simulink model "CCMVgaMotor"  (R2022b)
settings: Solver=FixedStepDiscrete  StartTime=0.0  StopTime=10.0  FixedStep=0.01  RelTol=1e-3
model workspace (MAT data in the model file):
  NvMBlockVgaMtrCalData : Simulink.Signal (value not decoded)
  NvMBlockVgaMtrCalDataInit : Simulink.Parameter (value not decoded)
  VgaWakeUpFlag : Simulink.Signal (value not decoded)
symbols that are objects in the model workspace (value not decoded): NvMBlockVgaMtrCalDataInit
undefined symbols (not defined in the file or in its folder): ComChlType, DioChannelType, LinFrameType, NetworkChlType, NvMBlockId, P_DioLevelType, P_LinIfSchHandleType, P_Std_ReturnType, PowerChlType, VGAChlType
(part 4 of 4)

== system CCMVgaMotor/VgaCaliServive ==
  VgaCaliServive_SetAllCalibrate_sys : Subsystem
  VgaCaliServive_SetAllParaInit_sys : Subsystem
  VgaCaliServive_SetMotorCalibrateNew_sys : Subsystem
  VgaCaliServive_SetMotorCalibrate_sys : Subsystem
  VgaCaliServive_SetMotorParaInit_sys : Subsystem

== system CCMVgaMotor/VgaDtcService ==
  VgaDtcService_GetElecErr_sys : Subsystem
  VgaDtcService_GetRecErr_sys : Subsystem
  VgaDtcService_GetStallErr_sys : Subsystem
  VgaDtcService_GetTempErr_sys : Subsystem
  VgaDtcService_GetVoltErr_sys : Subsystem

== system CCMVgaMotor/VgaIOCtrlService ==
  VgaIOCtrlService_FreezeMotorCurrentPosition_sys : Subsystem
  VgaIOCtrlService_MotorReturnToECU_sys : Subsystem
  VgaIOCtrlService_MotorShortTermAdj_sys : Subsystem

== system CCMVgaMotor/VgaNvmService ==
  VgaNvmService_ClerarNvmMotorData_sys : Subsystem

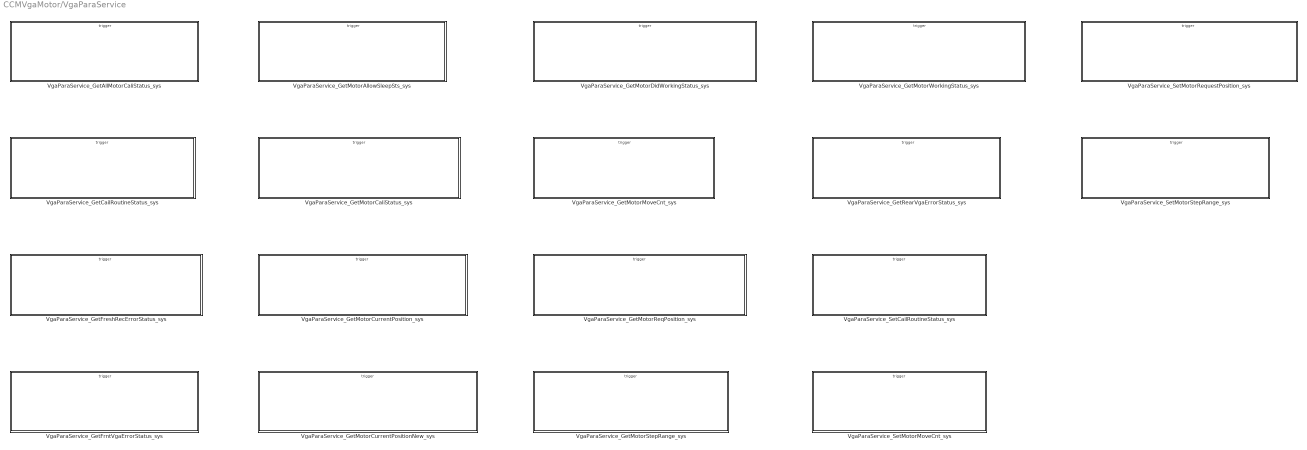
== system CCMVgaMotor/VgaParaService ==
  VgaParaService_GetAllMotorCaliStatus_sys : Subsystem
  VgaParaService_GetCailRoutineStatus_sys : Subsystem
  VgaParaService_GetFreshRecErrorStatus_sys : Subsystem
  VgaParaService_GetFrntVgaErrorStatus_sys : Subsystem
  VgaParaService_GetMotorAllowSleepSts_sys : Subsystem
  VgaParaService_GetMotorCaliStatus_sys : Subsystem
  VgaParaService_GetMotorCurrentPositionNew_sys : Subsystem
  VgaParaService_GetMotorCurrentPosition_sys : Subsystem
  VgaParaService_GetMotorDidWorkingStatus_sys : Subsystem
  VgaParaService_GetMotorMoveCnt_sys : Subsystem
  VgaParaService_GetMotorReqPosition_sys : Subsystem
  VgaParaService_GetMotorStepRange_sys : Subsystem
  VgaParaService_GetMotorWorkingStatus_sys : Subsystem
  VgaParaService_GetRearVgaErrorStatus_sys : Subsystem
  VgaParaService_SetCailRoutineStatus_sys : Subsystem
  VgaParaService_SetMotorMoveCnt_sys : Subsystem
  VgaParaService_SetMotorRequestPosition_sys : Subsystem
  VgaParaService_SetMotorStepRange_sys : Subsystem

== system CCMVgaMotor/VgaCaliServive/VgaCaliServive_SetAllCalibrate_sys ==
  function : TriggerPort  TriggerType=function-call  IsSimulinkFunction=on  FunctionName=VgaCaliServive_SetAllCalibrate  VariantControl=(inherit)
  Ground_1 : Ground
  PA_NvMBlockVgaMtrCalDataInit : Constant  Value=NvMBlockVgaMtrCalDataInit
  Terminator_1 : Terminator
  ERR : ArgOut  OutDataTypeStr=Std_ReturnType  ArgumentName=ERR
  wires:
  PA_NvMBlockVgaMtrCalDataInit/1 -> Terminator_1/1
  Ground_1/1 -> ERR/1

== system CCMVgaMotor/VgaCaliServive/VgaCaliServive_SetAllParaInit_sys ==
  function : TriggerPort  TriggerType=function-call  IsSimulinkFunction=on  FunctionName=VgaCaliServive_SetAllParaInit  VariantControl=(inherit)
  Ground_1 : Ground
  PA_NvMBlockVgaMtrCalDataInit : Constant  Value=NvMBlockVgaMtrCalDataInit
  Terminator_1 : Terminator
  ERR : ArgOut  OutDataTypeStr=Std_ReturnType  ArgumentName=ERR
  wires:
  PA_NvMBlockVgaMtrCalDataInit/1 -> Terminator_1/1
  Ground_1/1 -> ERR/1

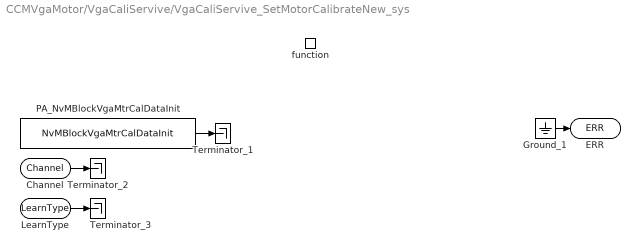
== system CCMVgaMotor/VgaCaliServive/VgaCaliServive_SetMotorCalibrateNew_sys ==
  function : TriggerPort  TriggerType=function-call  IsSimulinkFunction=on  FunctionName=VgaCaliServive_SetMotorCalibrateNew  VariantControl=(inherit)
  Ground_1 : Ground
  PA_NvMBlockVgaMtrCalDataInit : Constant  Value=NvMBlockVgaMtrCalDataInit
  Terminator_1 : Terminator
  Terminator_2 : Terminator
  Terminator_3 : Terminator
  Channel : ArgIn  OutDataTypeStr=Enum: VGAChlType  ArgumentName=Channel
  LearnType : ArgIn  Port=2  OutDataTypeStr=UInt8  ArgumentName=LearnType
  ERR : ArgOut  OutDataTypeStr=Std_ReturnType  ArgumentName=ERR
  wires:
  PA_NvMBlockVgaMtrCalDataInit/1 -> Terminator_1/1
  Channel/1 -> Terminator_2/1
  LearnType/1 -> Terminator_3/1
  Ground_1/1 -> ERR/1

== system CCMVgaMotor/VgaCaliServive/VgaCaliServive_SetMotorCalibrate_sys ==
  function : TriggerPort  TriggerType=function-call  IsSimulinkFunction=on  FunctionName=VgaCaliServive_SetMotorCalibrate  VariantControl=(inherit)
  Ground_1 : Ground
  PA_NvMBlockVgaMtrCalDataInit : Constant  Value=NvMBlockVgaMtrCalDataInit
  Terminator_1 : Terminator
  Terminator_2 : Terminator
  Channel : ArgIn  OutDataTypeStr=Enum: VGAChlType  ArgumentName=Channel
  ERR : ArgOut  OutDataTypeStr=Std_ReturnType  ArgumentName=ERR
  wires:
  PA_NvMBlockVgaMtrCalDataInit/1 -> Terminator_1/1
  Channel/1 -> Terminator_2/1
  Ground_1/1 -> ERR/1

== system CCMVgaMotor/VgaCaliServive/VgaCaliServive_SetMotorParaInit_sys ==
  function : TriggerPort  TriggerType=function-call  IsSimulinkFunction=on  FunctionName=VgaCaliServive_SetMotorParaInit  VariantControl=(inherit)
  Ground_1 : Ground
  PA_NvMBlockVgaMtrCalDataInit : Constant  Value=NvMBlockVgaMtrCalDataInit
  Terminator_1 : Terminator
  Terminator_2 : Terminator
  Channel : ArgIn  OutDataTypeStr=Enum: VGAChlType  ArgumentName=Channel
  ERR : ArgOut  OutDataTypeStr=Std_ReturnType  ArgumentName=ERR
  wires:
  PA_NvMBlockVgaMtrCalDataInit/1 -> Terminator_1/1
  Channel/1 -> Terminator_2/1
  Ground_1/1 -> ERR/1

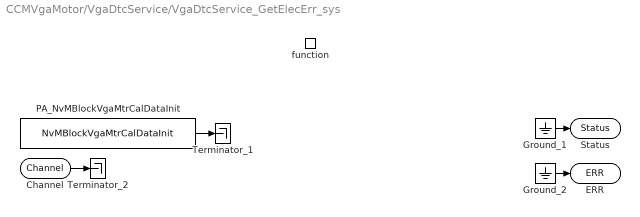
== system CCMVgaMotor/VgaDtcService/VgaDtcService_GetElecErr_sys ==
  function : TriggerPort  TriggerType=function-call  IsSimulinkFunction=on  FunctionName=VgaDtcService_GetElecErr  VariantControl=(inherit)
  Ground_1 : Ground
  Ground_2 : Ground
  PA_NvMBlockVgaMtrCalDataInit : Constant  Value=NvMBlockVgaMtrCalDataInit
  Terminator_1 : Terminator
  Terminator_2 : Terminator
  Channel : ArgIn  OutDataTypeStr=Enum: VGAChlType  ArgumentName=Channel
  Status : ArgOut  OutDataTypeStr=UInt8  ArgumentName=Status
  ERR : ArgOut  Port=2  OutDataTypeStr=Std_ReturnType  ArgumentName=ERR
  wires:
  PA_NvMBlockVgaMtrCalDataInit/1 -> Terminator_1/1
  Channel/1 -> Terminator_2/1
  Ground_1/1 -> Status/1
  Ground_2/1 -> ERR/1

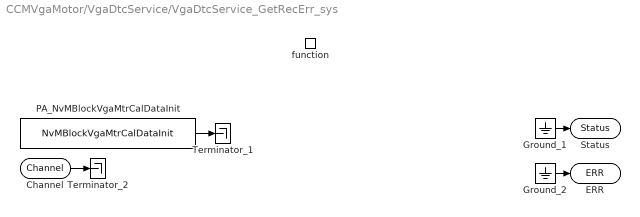
== system CCMVgaMotor/VgaDtcService/VgaDtcService_GetRecErr_sys ==
  function : TriggerPort  TriggerType=function-call  IsSimulinkFunction=on  FunctionName=VgaDtcService_GetRecErr  VariantControl=(inherit)
  Ground_1 : Ground
  Ground_2 : Ground
  PA_NvMBlockVgaMtrCalDataInit : Constant  Value=NvMBlockVgaMtrCalDataInit
  Terminator_1 : Terminator
  Terminator_2 : Terminator
  Channel : ArgIn  OutDataTypeStr=Enum: VGAChlType  ArgumentName=Channel
  Status : ArgOut  OutDataTypeStr=UInt8  ArgumentName=Status
  ERR : ArgOut  Port=2  OutDataTypeStr=Std_ReturnType  ArgumentName=ERR
  wires:
  PA_NvMBlockVgaMtrCalDataInit/1 -> Terminator_1/1
  Channel/1 -> Terminator_2/1
  Ground_1/1 -> Status/1
  Ground_2/1 -> ERR/1

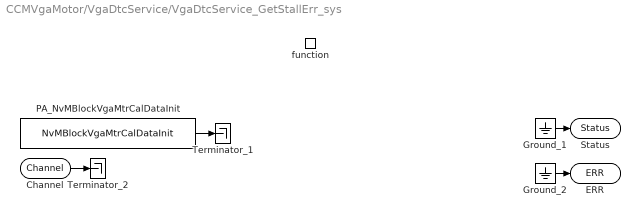
== system CCMVgaMotor/VgaDtcService/VgaDtcService_GetStallErr_sys ==
  function : TriggerPort  TriggerType=function-call  IsSimulinkFunction=on  FunctionName=VgaDtcService_GetStallErr  VariantControl=(inherit)
  Ground_1 : Ground
  Ground_2 : Ground
  PA_NvMBlockVgaMtrCalDataInit : Constant  Value=NvMBlockVgaMtrCalDataInit
  Terminator_1 : Terminator
  Terminator_2 : Terminator
  Channel : ArgIn  OutDataTypeStr=Enum: VGAChlType  ArgumentName=Channel
  Status : ArgOut  OutDataTypeStr=UInt8  ArgumentName=Status
  ERR : ArgOut  Port=2  OutDataTypeStr=Std_ReturnType  ArgumentName=ERR
  wires:
  PA_NvMBlockVgaMtrCalDataInit/1 -> Terminator_1/1
  Channel/1 -> Terminator_2/1
  Ground_1/1 -> Status/1
  Ground_2/1 -> ERR/1

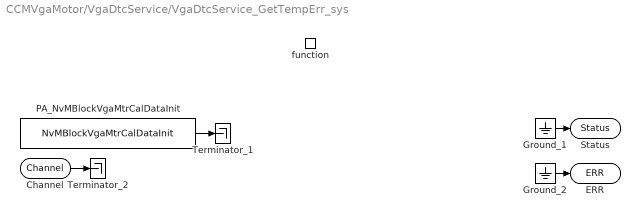
== system CCMVgaMotor/VgaDtcService/VgaDtcService_GetTempErr_sys ==
  function : TriggerPort  TriggerType=function-call  IsSimulinkFunction=on  FunctionName=VgaDtcService_GetTempErr  VariantControl=(inherit)
  Ground_1 : Ground
  Ground_2 : Ground
  PA_NvMBlockVgaMtrCalDataInit : Constant  Value=NvMBlockVgaMtrCalDataInit
  Terminator_1 : Terminator
  Terminator_2 : Terminator
  Channel : ArgIn  OutDataTypeStr=Enum: VGAChlType  ArgumentName=Channel
  Status : ArgOut  OutDataTypeStr=UInt8  ArgumentName=Status
  ERR : ArgOut  Port=2  OutDataTypeStr=Std_ReturnType  ArgumentName=ERR
  wires:
  PA_NvMBlockVgaMtrCalDataInit/1 -> Terminator_1/1
  Channel/1 -> Terminator_2/1
  Ground_1/1 -> Status/1
  Ground_2/1 -> ERR/1

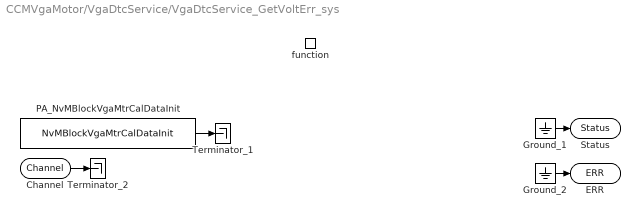
== system CCMVgaMotor/VgaDtcService/VgaDtcService_GetVoltErr_sys ==
  function : TriggerPort  TriggerType=function-call  IsSimulinkFunction=on  FunctionName=VgaDtcService_GetVoltErr  VariantControl=(inherit)
  Ground_1 : Ground
  Ground_2 : Ground
  PA_NvMBlockVgaMtrCalDataInit : Constant  Value=NvMBlockVgaMtrCalDataInit
  Terminator_1 : Terminator
  Terminator_2 : Terminator
  Channel : ArgIn  OutDataTypeStr=Enum: VGAChlType  ArgumentName=Channel
  Status : ArgOut  OutDataTypeStr=UInt8  ArgumentName=Status
  ERR : ArgOut  Port=2  OutDataTypeStr=Std_ReturnType  ArgumentName=ERR
  wires:
  PA_NvMBlockVgaMtrCalDataInit/1 -> Terminator_1/1
  Channel/1 -> Terminator_2/1
  Ground_1/1 -> Status/1
  Ground_2/1 -> ERR/1

== system CCMVgaMotor/VgaIOCtrlService/VgaIOCtrlService_FreezeMotorCurrentPosition_sys ==
  function : TriggerPort  TriggerType=function-call  IsSimulinkFunction=on  FunctionName=VgaIOCtrlService_FreezeMotorCurrentPosition  VariantControl=(inherit)
  Ground_1 : Ground
  PA_NvMBlockVgaMtrCalDataInit : Constant  Value=NvMBlockVgaMtrCalDataInit
  Terminator_1 : Terminator
  Terminator_2 : Terminator
  Channel : ArgIn  OutDataTypeStr=Enum: VGAChlType  ArgumentName=Channel
  ERR : ArgOut  OutDataTypeStr=Std_ReturnType  ArgumentName=ERR
  wires:
  PA_NvMBlockVgaMtrCalDataInit/1 -> Terminator_1/1
  Channel/1 -> Terminator_2/1
  Ground_1/1 -> ERR/1

== system CCMVgaMotor/VgaIOCtrlService/VgaIOCtrlService_MotorReturnToECU_sys ==
  function : TriggerPort  TriggerType=function-call  IsSimulinkFunction=on  FunctionName=VgaIOCtrlService_MotorReturnToECU  VariantControl=(inherit)
  Ground_1 : Ground
  PA_NvMBlockVgaMtrCalDataInit : Constant  Value=NvMBlockVgaMtrCalDataInit
  Terminator_1 : Terminator
  Terminator_2 : Terminator
  Channel : ArgIn  OutDataTypeStr=Enum: VGAChlType  ArgumentName=Channel
  ERR : ArgOut  OutDataTypeStr=Std_ReturnType  ArgumentName=ERR
  wires:
  PA_NvMBlockVgaMtrCalDataInit/1 -> Terminator_1/1
  Channel/1 -> Terminator_2/1
  Ground_1/1 -> ERR/1

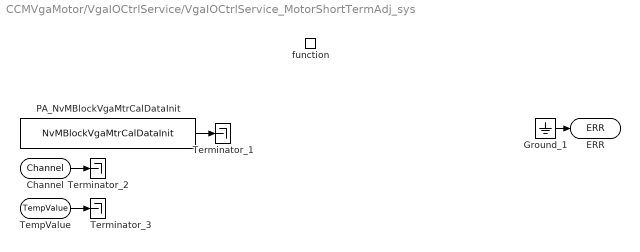
== system CCMVgaMotor/VgaIOCtrlService/VgaIOCtrlService_MotorShortTermAdj_sys ==
  function : TriggerPort  TriggerType=function-call  IsSimulinkFunction=on  FunctionName=VgaIOCtrlService_MotorShortTermAdj  VariantControl=(inherit)
  Ground_1 : Ground
  PA_NvMBlockVgaMtrCalDataInit : Constant  Value=NvMBlockVgaMtrCalDataInit
  Terminator_1 : Terminator
  Terminator_2 : Terminator
  Terminator_3 : Terminator
  Channel : ArgIn  OutDataTypeStr=Enum: VGAChlType  ArgumentName=Channel
  TempValue : ArgIn  Port=2  OutDataTypeStr=UInt16  ArgumentName=TempValue
  ERR : ArgOut  OutDataTypeStr=Std_ReturnType  ArgumentName=ERR
  wires:
  PA_NvMBlockVgaMtrCalDataInit/1 -> Terminator_1/1
  Channel/1 -> Terminator_2/1
  TempValue/1 -> Terminator_3/1
  Ground_1/1 -> ERR/1

== system CCMVgaMotor/VgaNvmService/VgaNvmService_ClerarNvmMotorData_sys ==
  function : TriggerPort  TriggerType=function-call  IsSimulinkFunction=on  FunctionName=VgaNvmService_ClerarNvmMotorData  VariantControl=(inherit)
  Ground_1 : Ground
  PA_NvMBlockVgaMtrCalDataInit : Constant  Value=NvMBlockVgaMtrCalDataInit
  Terminator_1 : Terminator
  ERR : ArgOut  OutDataTypeStr=Std_ReturnType  ArgumentName=ERR
  wires:
  PA_NvMBlockVgaMtrCalDataInit/1 -> Terminator_1/1
  Ground_1/1 -> ERR/1

== system CCMVgaMotor/VgaParaService/VgaParaService_GetAllMotorCaliStatus_sys ==
  function : TriggerPort  TriggerType=function-call  IsSimulinkFunction=on  FunctionName=VgaParaService_GetAllMotorCaliStatus  VariantControl=(inherit)
  Ground_1 : Ground
  Ground_2 : Ground
  PA_NvMBlockVgaMtrCalDataInit : Constant  Value=NvMBlockVgaMtrCalDataInit
  Terminator_1 : Terminator
  Status : ArgOut  OutDataTypeStr=UInt8  ArgumentName=Status
  ERR : ArgOut  Port=2  OutDataTypeStr=Std_ReturnType  ArgumentName=ERR
  wires:
  PA_NvMBlockVgaMtrCalDataInit/1 -> Terminator_1/1
  Ground_1/1 -> Status/1
  Ground_2/1 -> ERR/1

== system CCMVgaMotor/VgaParaService/VgaParaService_GetCailRoutineStatus_sys ==
  function : TriggerPort  TriggerType=function-call  IsSimulinkFunction=on  FunctionName=VgaParaService_GetCailRoutineStatus  VariantControl=(inherit)
  Ground_1 : Ground
  Ground_2 : Ground
  PA_NvMBlockVgaMtrCalDataInit : Constant  Value=NvMBlockVgaMtrCalDataInit
  Terminator_1 : Terminator
  Status : ArgOut  OutDataTypeStr=Enum: MtrRoutStaEnum  ArgumentName=Status
  ERR : ArgOut  Port=2  OutDataTypeStr=Std_ReturnType  ArgumentName=ERR
  wires:
  PA_NvMBlockVgaMtrCalDataInit/1 -> Terminator_1/1
  Ground_1/1 -> Status/1
  Ground_2/1 -> ERR/1

== system CCMVgaMotor/VgaParaService/VgaParaService_GetFreshRecErrorStatus_sys ==
  function : TriggerPort  TriggerType=function-call  IsSimulinkFunction=on  FunctionName=VgaParaService_GetFreshRecErrorStatus  VariantControl=(inherit)
  Ground_1 : Ground
  Ground_2 : Ground
  PA_NvMBlockVgaMtrCalDataInit : Constant  Value=NvMBlockVgaMtrCalDataInit
  Terminator_1 : Terminator
  Status : ArgOut  OutDataTypeStr=UInt8  ArgumentName=Status
  ERR : ArgOut  Port=2  OutDataTypeStr=Std_ReturnType  ArgumentName=ERR
  wires:
  PA_NvMBlockVgaMtrCalDataInit/1 -> Terminator_1/1
  Ground_1/1 -> Status/1
  Ground_2/1 -> ERR/1

== system CCMVgaMotor/VgaParaService/VgaParaService_GetFrntVgaErrorStatus_sys ==
  function : TriggerPort  TriggerType=function-call  IsSimulinkFunction=on  FunctionName=VgaParaService_GetFrntVgaErrorStatus  VariantControl=(inherit)
  Ground_1 : Ground
  Ground_2 : Ground
  PA_NvMBlockVgaMtrCalDataInit : Constant  Value=NvMBlockVgaMtrCalDataInit
  Terminator_1 : Terminator
  Status : ArgOut  OutDataTypeStr=UInt8  ArgumentName=Status
  ERR : ArgOut  Port=2  OutDataTypeStr=Std_ReturnType  ArgumentName=ERR
  wires:
  PA_NvMBlockVgaMtrCalDataInit/1 -> Terminator_1/1
  Ground_1/1 -> Status/1
  Ground_2/1 -> ERR/1

== system CCMVgaMotor/VgaParaService/VgaParaService_GetMotorAllowSleepSts_sys ==
  function : TriggerPort  TriggerType=function-call  IsSimulinkFunction=on  FunctionName=VgaParaService_GetMotorAllowSleepSts  VariantControl=(inherit)
  Ground_1 : Ground
  Ground_2 : Ground
  PA_NvMBlockVgaMtrCalDataInit : Constant  Value=NvMBlockVgaMtrCalDataInit
  Terminator_1 : Terminator
  Status : ArgOut  OutDataTypeStr=UInt8  ArgumentName=Status
  ERR : ArgOut  Port=2  OutDataTypeStr=Std_ReturnType  ArgumentName=ERR
  wires:
  PA_NvMBlockVgaMtrCalDataInit/1 -> Terminator_1/1
  Ground_1/1 -> Status/1
  Ground_2/1 -> ERR/1

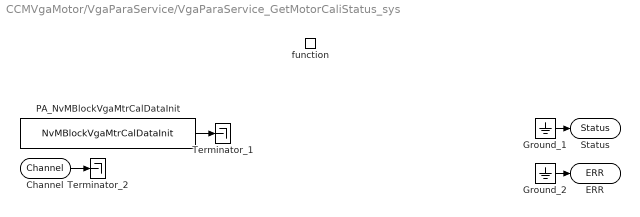
== system CCMVgaMotor/VgaParaService/VgaParaService_GetMotorCaliStatus_sys ==
  function : TriggerPort  TriggerType=function-call  IsSimulinkFunction=on  FunctionName=VgaParaService_GetMotorCaliStatus  VariantControl=(inherit)
  Ground_1 : Ground
  Ground_2 : Ground
  PA_NvMBlockVgaMtrCalDataInit : Constant  Value=NvMBlockVgaMtrCalDataInit
  Terminator_1 : Terminator
  Terminator_2 : Terminator
  Channel : ArgIn  OutDataTypeStr=Enum: VGAChlType  ArgumentName=Channel
  Status : ArgOut  OutDataTypeStr=UInt8  ArgumentName=Status
  ERR : ArgOut  Port=2  OutDataTypeStr=Std_ReturnType  ArgumentName=ERR
  wires:
  PA_NvMBlockVgaMtrCalDataInit/1 -> Terminator_1/1
  Channel/1 -> Terminator_2/1
  Ground_1/1 -> Status/1
  Ground_2/1 -> ERR/1

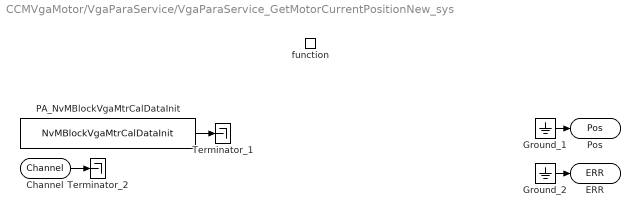
== system CCMVgaMotor/VgaParaService/VgaParaService_GetMotorCurrentPositionNew_sys ==
  function : TriggerPort  TriggerType=function-call  IsSimulinkFunction=on  FunctionName=VgaParaService_GetMotorCurrentPositionNew  VariantControl=(inherit)
  Ground_1 : Ground
  Ground_2 : Ground
  PA_NvMBlockVgaMtrCalDataInit : Constant  Value=NvMBlockVgaMtrCalDataInit
  Terminator_1 : Terminator
  Terminator_2 : Terminator
  Channel : ArgIn  OutDataTypeStr=Enum: VGAChlType  ArgumentName=Channel
  Pos : ArgOut  OutDataTypeStr=UInt16  ArgumentName=Pos
  ERR : ArgOut  Port=2  OutDataTypeStr=Std_ReturnType  ArgumentName=ERR
  wires:
  PA_NvMBlockVgaMtrCalDataInit/1 -> Terminator_1/1
  Channel/1 -> Terminator_2/1
  Ground_1/1 -> Pos/1
  Ground_2/1 -> ERR/1

== system CCMVgaMotor/VgaParaService/VgaParaService_GetMotorCurrentPosition_sys ==
  function : TriggerPort  TriggerType=function-call  IsSimulinkFunction=on  FunctionName=VgaParaService_GetMotorCurrentPosition  VariantControl=(inherit)
  Ground_1 : Ground
  Ground_2 : Ground
  PA_NvMBlockVgaMtrCalDataInit : Constant  Value=NvMBlockVgaMtrCalDataInit
  Terminator_1 : Terminator
  Terminator_2 : Terminator
  Channel : ArgIn  OutDataTypeStr=Enum: VGAChlType  ArgumentName=Channel
  Pos : ArgOut  OutDataTypeStr=UInt8  ArgumentName=Pos
  ERR : ArgOut  Port=2  OutDataTypeStr=Std_ReturnType  ArgumentName=ERR
  wires:
  PA_NvMBlockVgaMtrCalDataInit/1 -> Terminator_1/1
  Channel/1 -> Terminator_2/1
  Ground_1/1 -> Pos/1
  Ground_2/1 -> ERR/1

== system CCMVgaMotor/VgaParaService/VgaParaService_GetMotorDidWorkingStatus_sys ==
  function : TriggerPort  TriggerType=function-call  IsSimulinkFunction=on  FunctionName=VgaParaService_GetMotorDidWorkingStatus  VariantControl=(inherit)
  Ground_1 : Ground
  Ground_2 : Ground
  PA_NvMBlockVgaMtrCalDataInit : Constant  Value=NvMBlockVgaMtrCalDataInit
  Terminator_1 : Terminator
  Terminator_2 : Terminator
  Channel : ArgIn  OutDataTypeStr=Enum: VGAChlType  ArgumentName=Channel
  Status : ArgOut  OutDataTypeStr=UInt8  ArgumentName=Status
  ERR : ArgOut  Port=2  OutDataTypeStr=Std_ReturnType  ArgumentName=ERR
  wires:
  PA_NvMBlockVgaMtrCalDataInit/1 -> Terminator_1/1
  Channel/1 -> Terminator_2/1
  Ground_1/1 -> Status/1
  Ground_2/1 -> ERR/1

== system CCMVgaMotor/VgaParaService/VgaParaService_GetMotorMoveCnt_sys ==
  function : TriggerPort  TriggerType=function-call  IsSimulinkFunction=on  FunctionName=VgaParaService_GetMotorMoveCnt  VariantControl=(inherit)
  Ground_1 : Ground
  Ground_2 : Ground
  PA_NvMBlockVgaMtrCalDataInit : Constant  Value=NvMBlockVgaMtrCalDataInit
  Terminator_1 : Terminator
  Terminator_2 : Terminator
  Channel : ArgIn  OutDataTypeStr=Enum: VGAChlType  ArgumentName=Channel
  Cnt : ArgOut  OutDataTypeStr=UInt32  ArgumentName=Cnt
  ERR : ArgOut  Port=2  OutDataTypeStr=Std_ReturnType  ArgumentName=ERR
  wires:
  PA_NvMBlockVgaMtrCalDataInit/1 -> Terminator_1/1
  Channel/1 -> Terminator_2/1
  Ground_1/1 -> Cnt/1
  Ground_2/1 -> ERR/1

== system CCMVgaMotor/VgaParaService/VgaParaService_GetMotorReqPosition_sys ==
  function : TriggerPort  TriggerType=function-call  IsSimulinkFunction=on  FunctionName=VgaParaService_GetMotorReqPosition  VariantControl=(inherit)
  Ground_1 : Ground
  Ground_2 : Ground
  PA_NvMBlockVgaMtrCalDataInit : Constant  Value=NvMBlockVgaMtrCalDataInit
  Terminator_1 : Terminator
  Terminator_2 : Terminator
  Channel : ArgIn  OutDataTypeStr=Enum: VGAChlType  ArgumentName=Channel
  Position : ArgOut  OutDataTypeStr=UInt8  ArgumentName=Position
  ERR : ArgOut  Port=2  OutDataTypeStr=Std_ReturnType  ArgumentName=ERR
  wires:
  PA_NvMBlockVgaMtrCalDataInit/1 -> Terminator_1/1
  Channel/1 -> Terminator_2/1
  Ground_1/1 -> Position/1
  Ground_2/1 -> ERR/1

== system CCMVgaMotor/VgaParaService/VgaParaService_GetMotorStepRange_sys ==
  function : TriggerPort  TriggerType=function-call  IsSimulinkFunction=on  FunctionName=VgaParaService_GetMotorStepRange  VariantControl=(inherit)
  Ground_1 : Ground
  Ground_2 : Ground
  PA_NvMBlockVgaMtrCalDataInit : Constant  Value=NvMBlockVgaMtrCalDataInit
  Terminator_1 : Terminator
  Terminator_2 : Terminator
  Channel : ArgIn  OutDataTypeStr=Enum: VGAChlType  ArgumentName=Channel
  Range : ArgOut  OutDataTypeStr=UInt16  ArgumentName=Range
  ERR : ArgOut  Port=2  OutDataTypeStr=Std_ReturnType  ArgumentName=ERR
  wires:
  PA_NvMBlockVgaMtrCalDataInit/1 -> Terminator_1/1
  Channel/1 -> Terminator_2/1
  Ground_1/1 -> Range/1
  Ground_2/1 -> ERR/1

== system CCMVgaMotor/VgaParaService/VgaParaService_GetMotorWorkingStatus_sys ==
  function : TriggerPort  TriggerType=function-call  IsSimulinkFunction=on  FunctionName=VgaParaService_GetMotorWorkingStatus  VariantControl=(inherit)
  Ground_1 : Ground
  Ground_2 : Ground
  PA_NvMBlockVgaMtrCalDataInit : Constant  Value=NvMBlockVgaMtrCalDataInit
  Terminator_1 : Terminator
  Terminator_2 : Terminator
  Channel : ArgIn  OutDataTypeStr=Enum: VGAChlType  ArgumentName=Channel
  Status : ArgOut  OutDataTypeStr=UInt8  ArgumentName=Status
  ERR : ArgOut  Port=2  OutDataTypeStr=Std_ReturnType  ArgumentName=ERR
  wires:
  PA_NvMBlockVgaMtrCalDataInit/1 -> Terminator_1/1
  Channel/1 -> Terminator_2/1
  Ground_1/1 -> Status/1
  Ground_2/1 -> ERR/1

== system CCMVgaMotor/VgaParaService/VgaParaService_GetRearVgaErrorStatus_sys ==
  function : TriggerPort  TriggerType=function-call  IsSimulinkFunction=on  FunctionName=VgaParaService_GetRearVgaErrorStatus  VariantControl=(inherit)
  Ground_1 : Ground
  Ground_2 : Ground
  PA_NvMBlockVgaMtrCalDataInit : Constant  Value=NvMBlockVgaMtrCalDataInit
  Terminator_1 : Terminator
  Status : ArgOut  OutDataTypeStr=UInt8  ArgumentName=Status
  ERR : ArgOut  Port=2  OutDataTypeStr=Std_ReturnType  ArgumentName=ERR
  wires:
  PA_NvMBlockVgaMtrCalDataInit/1 -> Terminator_1/1
  Ground_1/1 -> Status/1
  Ground_2/1 -> ERR/1

== system CCMVgaMotor/VgaParaService/VgaParaService_SetCailRoutineStatus_sys ==
  function : TriggerPort  TriggerType=function-call  IsSimulinkFunction=on  FunctionName=VgaParaService_SetCailRoutineStatus  VariantControl=(inherit)
  Ground_1 : Ground
  PA_NvMBlockVgaMtrCalDataInit : Constant  Value=NvMBlockVgaMtrCalDataInit
  Terminator_1 : Terminator
  Terminator_2 : Terminator
  Status : ArgIn  OutDataTypeStr=Enum: MtrRoutStaEnum  ArgumentName=Status
  ERR : ArgOut  OutDataTypeStr=Std_ReturnType  ArgumentName=ERR
  wires:
  PA_NvMBlockVgaMtrCalDataInit/1 -> Terminator_1/1
  Status/1 -> Terminator_2/1
  Ground_1/1 -> ERR/1

== system CCMVgaMotor/VgaParaService/VgaParaService_SetMotorMoveCnt_sys ==
  function : TriggerPort  TriggerType=function-call  IsSimulinkFunction=on  FunctionName=VgaParaService_SetMotorMoveCnt  VariantControl=(inherit)
  Ground_1 : Ground
  PA_NvMBlockVgaMtrCalDataInit : Constant  Value=NvMBlockVgaMtrCalDataInit
  Terminator_1 : Terminator
  Terminator_2 : Terminator
  Terminator_3 : Terminator
  Channel : ArgIn  OutDataTypeStr=Enum: VGAChlType  ArgumentName=Channel
  Cnt : ArgIn  Port=2  OutDataTypeStr=UInt32  ArgumentName=Cnt
  ERR : ArgOut  OutDataTypeStr=Std_ReturnType  ArgumentName=ERR
  wires:
  PA_NvMBlockVgaMtrCalDataInit/1 -> Terminator_1/1
  Channel/1 -> Terminator_2/1
  Cnt/1 -> Terminator_3/1
  Ground_1/1 -> ERR/1

== system CCMVgaMotor/VgaParaService/VgaParaService_SetMotorRequestPosition_sys ==
  function : TriggerPort  TriggerType=function-call  IsSimulinkFunction=on  FunctionName=VgaParaService_SetMotorRequestPosition  VariantControl=(inherit)
  Ground_1 : Ground
  PA_NvMBlockVgaMtrCalDataInit : Constant  Value=NvMBlockVgaMtrCalDataInit
  Terminator_1 : Terminator
  Terminator_2 : Terminator
  Terminator_3 : Terminator
  Channel : ArgIn  OutDataTypeStr=Enum: VGAChlType  ArgumentName=Channel
  Present : ArgIn  Port=2  OutDataTypeStr=UInt16  ArgumentName=Present
  ERR : ArgOut  OutDataTypeStr=Std_ReturnType  ArgumentName=ERR
  wires:
  PA_NvMBlockVgaMtrCalDataInit/1 -> Terminator_1/1
  Channel/1 -> Terminator_2/1
  Present/1 -> Terminator_3/1
  Ground_1/1 -> ERR/1

== system CCMVgaMotor/VgaParaService/VgaParaService_SetMotorStepRange_sys ==
  function : TriggerPort  TriggerType=function-call  IsSimulinkFunction=on  FunctionName=VgaParaService_SetMotorStepRange  VariantControl=(inherit)
  Ground_1 : Ground
  PA_NvMBlockVgaMtrCalDataInit : Constant  Value=NvMBlockVgaMtrCalDataInit
  Terminator_1 : Terminator
  Terminator_2 : Terminator
  Terminator_3 : Terminator
  Channel : ArgIn  OutDataTypeStr=Enum: VGAChlType  ArgumentName=Channel
  Range : ArgIn  Port=2  OutDataTypeStr=UInt16  ArgumentName=Range
  ERR : ArgOut  OutDataTypeStr=Std_ReturnType  ArgumentName=ERR
  wires:
  PA_NvMBlockVgaMtrCalDataInit/1 -> Terminator_1/1
  Channel/1 -> Terminator_2/1
  Range/1 -> Terminator_3/1
  Ground_1/1 -> ERR/1
